simulink model "HvacMdlAirTDistbnStatFildFrnt"  (R2022b)
settings: Solver=FixedStepDiscrete  StartTime=0.0  StopTime=10.0  FixedStep=0.2  RelTol=1e-3
settings Model: Creator=blue.ge  LastModifiedBy=blue.ge
settings DataIOCC: SaveFormat=StructureWithTime
settings OptimizationCC: DefaultParameterBehavior=Inlined  EnhancedBackFolding=on  PassReuseOutputArgsAs=Individual arguments  ZeroExternalMemoryAtStartup=off  ZeroInternalMemoryAtStartup=off  OptimizeBlockOrder=speed  DifferentSizesBufferReuse=on  OptimizationCustomize=off  EfficientTunableParamExpr=on
settings DebuggingCC: ParameterTunabilityLossMsg=error
settings ModelReferenceCC: ModelReferenceNumInstancesAllowed=Single
settings SFSimCC: MATLABDynamicMemAlloc=off
settings RTWCC: Description=AUTOSAR  SystemTargetFile=autosar.tlc  GenCodeOnly=on  TemplateMakefile=ert_default_tmf
settings CodeAppCC: ForceParamTrailComments=on  IgnoreCustomStorageClasses=off  OperatorAnnotations=on  SimulinkDataObjDesc=on  SFDataObjDesc=on  InsertBlockDesc=on
settings STFCustomTargetCC: TargetLangStandard=C99 (ISO)  MultiwordLength=256  ParMdlRefBuildCompliant=on  CompOptLevelCompliant=on  IncludeMdlTerminateFcn=off  CombineOutputUpdateFcns=on  SuppressErrorStatus=on  ERTCustomFileBanners=on  SupportNonFinite=off  SupportComplex=off  SupportContinuousTime=off  SupportNonInlinedSFcns=off  RemoveResetFunc=on  MATLABClassNameForMDSCustomization=Simulink.SoftwareTarget.ERTCustomization  AutosarCompliant=on  UseToolchainInfoCompliant=on  LookupTableObjectStructAxisOrder=2,1,3,4,...  LUTObjectStructOrderEvenSpacing=Size,Table,Breakpoints  SystemTargetFile=autosar.tlc
settings ERTTargetCC: TargetLangStandard=C99 (ISO)  ConcurrentExecutionCompliant=off  IncludeMdlTerminateFcn=off  SuppressErrorStatus=on  SupportNonFinite=off  SupportComplex=off  ModelStepFunctionPrototypeControlCompliant=off  CPPClassGenCompliant=off  AutosarCompliant=on  LookupTableObjectStructAxisOrder=2,1,3,4,...  LUTObjectStructOrderEvenSpacing=Size,Table,Breakpoints  InlinedParameterPlacement=NonHierarchical  ERTCustomFileTemplate=example_file_process.tlc

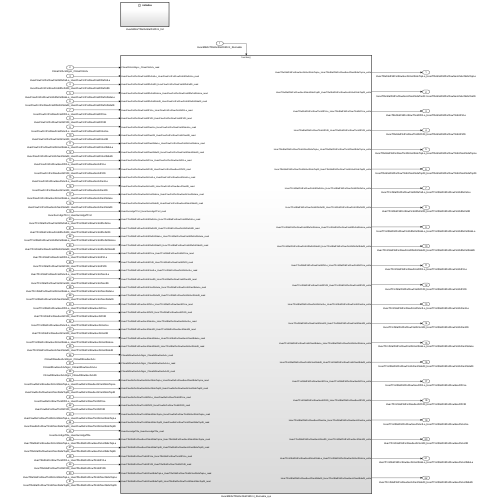
[thumbnail: Simulink's stored preview of the top-level diagram]
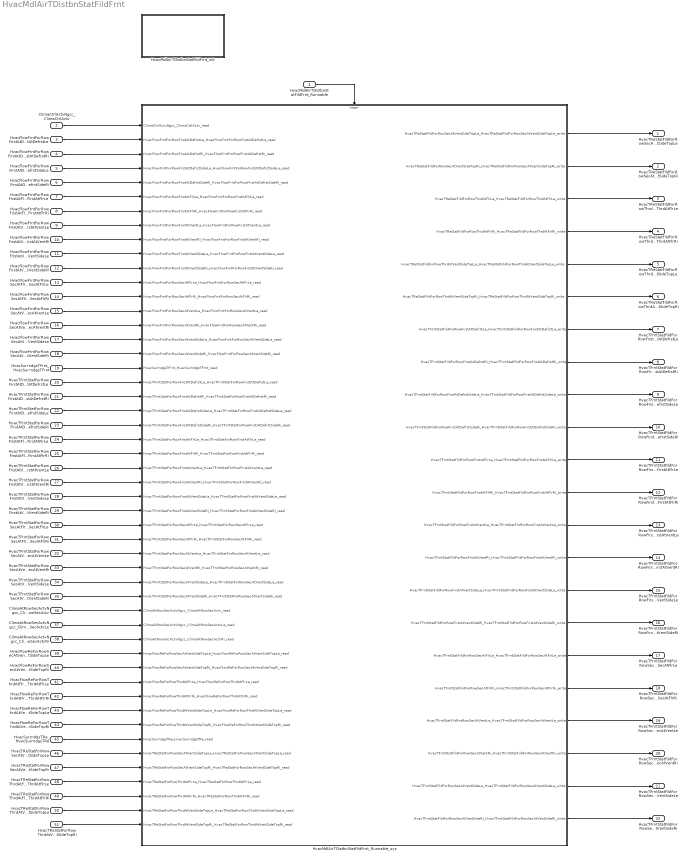
== system HvacMdlAirTDistbnStatFildFrnt ==
  HvacMdlAirTDistbnStatFildFrnt_Runnable : Inport  SampleTime=0.2  OutputFunctionCall=on
  ClimaCtrlActvNgcc_ClimaCtrlActv : Inport  Port=2  OutDataTypeStr=Boolean  PortDimensions=1
  HvacFlowFrntForRowFirstAtDefrstLe_HvacFlowFrntForRowFirstAtDefrstLe : Inport  Port=3  OutDataTypeStr=AirFlow30  PortDimensions=1
  HvacFlowFrntForRowFirstAtDefrstRi_HvacFlowFrntForRowFirstAtDefrstRi : Inport  Port=4  OutDataTypeStr=AirFlow30  PortDimensions=1
  HvacFlowFrntForRowFirstAtDefrstSideLe_HvacFlowFrntForRowFirstAtDefrstSideLe : Inport  Port=5  OutDataTypeStr=AirFlow30  PortDimensions=1
  HvacFlowFrntForRowFirstAtDefrstSideRi_HvacFlowFrntForRowFirstAtDefrstSideRi : Inport  Port=6  OutDataTypeStr=AirFlow30  PortDimensions=1
  HvacFlowFrntForRowFirstAtFlrLe_HvacFlowFrntForRowFirstAtFlrLe : Inport  Port=7  OutDataTypeStr=AirFlow30  PortDimensions=1
  HvacFlowFrntForRowFirstAtFlrRi_HvacFlowFrntForRowFirstAtFlrRi : Inport  Port=8  OutDataTypeStr=AirFlow30  PortDimensions=1
  HvacFlowFrntForRowFirstAtVentLe_HvacFlowFrntForRowFirstAtVentLe : Inport  Port=9  OutDataTypeStr=AirFlow30  PortDimensions=1
  HvacFlowFrntForRowFirstAtVentRi_HvacFlowFrntForRowFirstAtVentRi : Inport  Port=10  OutDataTypeStr=AirFlow30  PortDimensions=1
  HvacFlowFrntForRowFirstAtVentSideLe_HvacFlowFrntForRowFirstAtVentSideLe : Inport  Port=11  OutDataTypeStr=AirFlow30  PortDimensions=1
  HvacFlowFrntForRowFirstAtVentSideRi_HvacFlowFrntForRowFirstAtVentSideRi : Inport  Port=12  OutDataTypeStr=AirFlow30  PortDimensions=1
  HvacFlowFrntForRowSecAtFlrLe_HvacFlowFrntForRowSecAtFlrLe : Inport  Port=13  OutDataTypeStr=AirFlow30  PortDimensions=1
  HvacFlowFrntForRowSecAtFlrRi_HvacFlowFrntForRowSecAtFlrRi : Inport  Port=14  OutDataTypeStr=AirFlow30  PortDimensions=1
  HvacFlowFrntForRowSecAtVentLe_HvacFlowFrntForRowSecAtVentLe : Inport  Port=15  OutDataTypeStr=AirFlow30  PortDimensions=1
  HvacFlowFrntForRowSecAtVentRi_HvacFlowFrntForRowSecAtVentRi : Inport  Port=16  OutDataTypeStr=AirFlow30  PortDimensions=1
  HvacFlowFrntForRowSecAtVentSideLe_HvacFlowFrntForRowSecAtVentSideLe : Inport  Port=17  OutDataTypeStr=AirFlow30  PortDimensions=1
  HvacFlowFrntForRowSecAtVentSideRi_HvacFlowFrntForRowSecAtVentSideRi : Inport  Port=18  OutDataTypeStr=AirFlow30  PortDimensions=1
  HvacSurrndgsTFrnt_HvacSurrndgsTFrnt : Inport  Port=19  OutDataTypeStr=T30  PortDimensions=1
  HvacTFrntStatForRowFirstAtDefrstLe_HvacTFrntStatForRowFirstAtDefrstLe : Inport  Port=20  OutDataTypeStr=T30  PortDimensions=1
  HvacTFrntStatForRowFirstAtDefrstRi_HvacTFrntStatForRowFirstAtDefrstRi : Inport  Port=21  OutDataTypeStr=T30  PortDimensions=1
  HvacTFrntStatForRowFirstAtDefrstSideLe_HvacTFrntStatForRowFirstAtDefrstSideLe : Inport  Port=22  OutDataTypeStr=T30  PortDimensions=1
  HvacTFrntStatForRowFirstAtDefrstSideRi_HvacTFrntStatForRowFirstAtDefrstSideRi : Inport  Port=23  OutDataTypeStr=T30  PortDimensions=1
  HvacTFrntStatForRowFirstAtFlrLe_HvacTFrntStatForRowFirstAtFlrLe : Inport  Port=24  OutDataTypeStr=T30  PortDimensions=1
  HvacTFrntStatForRowFirstAtFlrRi_HvacTFrntStatForRowFirstAtFlrRi : Inport  Port=25  OutDataTypeStr=T30  PortDimensions=1
  HvacTFrntStatForRowFirstAtVentLe_HvacTFrntStatForRowFirstAtVentLe : Inport  Port=26  OutDataTypeStr=T30  PortDimensions=1
  HvacTFrntStatForRowFirstAtVentRi_HvacTFrntStatForRowFirstAtVentRi : Inport  Port=27  OutDataTypeStr=T30  PortDimensions=1
  HvacTFrntStatForRowFirstAtVentSideLe_HvacTFrntStatForRowFirstAtVentSideLe : Inport  Port=28  OutDataTypeStr=T30  PortDimensions=1
  HvacTFrntStatForRowFirstAtVentSideRi_HvacTFrntStatForRowFirstAtVentSideRi : Inport  Port=29  OutDataTypeStr=T30  PortDimensions=1
  HvacTFrntStatForRowSecAtFlrLe_HvacTFrntStatForRowSecAtFlrLe : Inport  Port=30  OutDataTypeStr=T30  PortDimensions=1
  HvacTFrntStatForRowSecAtFlrRi_HvacTFrntStatForRowSecAtFlrRi : Inport  Port=31  OutDataTypeStr=T30  PortDimensions=1
  HvacTFrntStatForRowSecAtVentLe_HvacTFrntStatForRowSecAtVentLe : Inport  Port=32  OutDataTypeStr=T30  PortDimensions=1
  HvacTFrntStatForRowSecAtVentRi_HvacTFrntStatForRowSecAtVentRi : Inport  Port=33  OutDataTypeStr=T30  PortDimensions=1
  HvacTFrntStatForRowSecAtVentSideLe_HvacTFrntStatForRowSecAtVentSideLe : Inport  Port=34  OutDataTypeStr=T30  PortDimensions=1
  HvacTFrntStatForRowSecAtVentSideRi_HvacTFrntStatForRowSecAtVentSideRi : Inport  Port=35  OutDataTypeStr=T30  PortDimensions=1
  ClimaAtRowSecActvNgcc_ClimaAtRowSecActv : Inport  Port=36  OutDataTypeStr=Boolean  PortDimensions=1
  ClimaAtRowSecActvNgcc_ClimaAtRowSecActvLe : Inport  Port=37  OutDataTypeStr=Boolean  PortDimensions=1
  ClimaAtRowSecActvNgcc_ClimaAtRowSecActvRi : Inport  Port=38  OutDataTypeStr=Boolean  PortDimensions=1
  HvacFlowReForRowSecAtVentSideTopLe_HvacFlowReForRowSecAtVentSideTopLe : Inport  Port=39  OutDataTypeStr=AirFlow30  PortDimensions=1
  HvacFlowReForRowSecAtVentSideTopRi_HvacFlowReForRowSecAtVentSideTopRi : Inport  Port=40  OutDataTypeStr=AirFlow30  PortDimensions=1
  HvacFlowReForRowThrdAtFlrLe_HvacFlowReForRowThrdAtFlrLe : Inport  Port=41  OutDataTypeStr=AirFlow30  PortDimensions=1
  HvacFlowReForRowThrdAtFlrRi_HvacFlowReForRowThrdAtFlrRi : Inport  Port=42  OutDataTypeStr=AirFlow30  PortDimensions=1
  HvacFlowReForRowThrdAtVentSideTopLe_HvacFlowReForRowThrdAtVentSideTopLe : Inport  Port=43  OutDataTypeStr=AirFlow30  PortDimensions=1
  HvacFlowReForRowThrdAtVentSideTopRi_HvacFlowReForRowThrdAtVentSideTopRi : Inport  Port=44  OutDataTypeStr=AirFlow30  PortDimensions=1
  HvacSurrndgsTRe_HvacSurrndgsTRe : Inport  Port=45  OutDataTypeStr=T30  PortDimensions=1
  HvacTReStatForRowSecAtVentSideTopLe_HvacTReStatForRowSecAtVentSideTopLe : Inport  Port=46  OutDataTypeStr=T30  PortDimensions=1
  HvacTReStatForRowSecAtVentSideTopRi_HvacTReStatForRowSecAtVentSideTopRi : Inport  Port=47  OutDataTypeStr=T30  PortDimensions=1
  HvacTReStatForRowThrdAtFlrLe_HvacTReStatForRowThrdAtFlrLe : Inport  Port=48  OutDataTypeStr=T30  PortDimensions=1
  HvacTReStatForRowThrdAtFlrRi_HvacTReStatForRowThrdAtFlrRi : Inport  Port=49  OutDataTypeStr=T30  PortDimensions=1
  HvacTReStatForRowThrdAtVentSideTopLe_HvacTReStatForRowThrdAtVentSideTopLe : Inport  Port=50  OutDataTypeStr=T30  PortDimensions=1
  HvacTReStatForRowThrdAtVentSideTopRi_HvacTReStatForRowThrdAtVentSideTopRi : Inport  Port=51  OutDataTypeStr=T30  PortDimensions=1
  HvacMdlAirTDistbnStatFildFrnt_Init : Subsystem
  HvacMdlAirTDistbnStatFildFrnt_Runnable_sys : Subsystem
  HvacTReStatFildForRowSecAtVentSideTopLe_HvacTReStatFildForRowSecAtVentSideTopLe : Outport  OutDataTypeStr=T30  PortDimensions=1
  HvacTReStatFildForRowSecAtVentSideTopRi_HvacTReStatFildForRowSecAtVentSideTopRi : Outport  Port=2  OutDataTypeStr=T30  PortDimensions=1
  HvacTReStatFildForRowThrdAtFlrLe_HvacTReStatFildForRowThrdAtFlrLe : Outport  Port=3  OutDataTypeStr=T30  PortDimensions=1
  HvacTReStatFildForRowThrdAtFlrRi_HvacTReStatFildForRowThrdAtFlrRi : Outport  Port=4  OutDataTypeStr=T30  PortDimensions=1
  HvacTReStatFildForRowThrdAtVentSideTopLe_HvacTReStatFildForRowThrdAtVentSideTopLe : Outport  Port=5  OutDataTypeStr=T30  PortDimensions=1
  HvacTReStatFildForRowThrdAtVentSideTopRi_HvacTReStatFildForRowThrdAtVentSideTopRi : Outport  Port=6  OutDataTypeStr=T30  PortDimensions=1
  HvacTFrntStatFildForRowFirstAtDefrstLe_HvacTFrntStatFildForRowFirstAtDefrstLe : Outport  Port=7  OutDataTypeStr=T30  PortDimensions=1
  HvacTFrntStatFildForRowFirstAtDefrstRi_HvacTFrntStatFildForRowFirstAtDefrstRi : Outport  Port=8  OutDataTypeStr=T30  PortDimensions=1
  HvacTFrntStatFildForRowFirstAtDefrstSideLe_HvacTFrntStatFildForRowFirstAtDefrstSideLe : Outport  Port=9  OutDataTypeStr=T30  PortDimensions=1
  HvacTFrntStatFildForRowFirstAtDefrstSideRi_HvacTFrntStatFildForRowFirstAtDefrstSideRi : Outport  Port=10  OutDataTypeStr=T30  PortDimensions=1
  HvacTFrntStatFildForRowFirstAtFlrLe_HvacTFrntStatFildForRowFirstAtFlrLe : Outport  Port=11  OutDataTypeStr=T30  PortDimensions=1
  HvacTFrntStatFildForRowFirstAtFlrRi_HvacTFrntStatFildForRowFirstAtFlrRi : Outport  Port=12  OutDataTypeStr=T30  PortDimensions=1
  HvacTFrntStatFildForRowFirstAtVentLe_HvacTFrntStatFildForRowFirstAtVentLe : Outport  Port=13  OutDataTypeStr=T30  PortDimensions=1
  HvacTFrntStatFildForRowFirstAtVentRi_HvacTFrntStatFildForRowFirstAtVentRi : Outport  Port=14  OutDataTypeStr=T30  PortDimensions=1
  HvacTFrntStatFildForRowFirstAtVentSideLe_HvacTFrntStatFildForRowFirstAtVentSideLe : Outport  Port=15  OutDataTypeStr=T30  PortDimensions=1
  HvacTFrntStatFildForRowFirstAtVentSideRi_HvacTFrntStatFildForRowFirstAtVentSideRi : Outport  Port=16  OutDataTypeStr=T30  PortDimensions=1
  HvacTFrntStatFildForRowSecAtFlrLe_HvacTFrntStatFildForRowSecAtFlrLe : Outport  Port=17  OutDataTypeStr=T30  PortDimensions=1
  HvacTFrntStatFildForRowSecAtFlrRi_HvacTFrntStatFildForRowSecAtFlrRi : Outport  Port=18  OutDataTypeStr=T30  PortDimensions=1
  HvacTFrntStatFildForRowSecAtVentLe_HvacTFrntStatFildForRowSecAtVentLe : Outport  Port=19  OutDataTypeStr=T30  PortDimensions=1
  HvacTFrntStatFildForRowSecAtVentRi_HvacTFrntStatFildForRowSecAtVentRi : Outport  Port=20  OutDataTypeStr=T30  PortDimensions=1
  HvacTFrntStatFildForRowSecAtVentSideLe_HvacTFrntStatFildForRowSecAtVentSideLe : Outport  Port=21  OutDataTypeStr=T30  PortDimensions=1
  HvacTFrntStatFildForRowSecAtVentSideRi_HvacTFrntStatFildForRowSecAtVentSideRi : Outport  Port=22  OutDataTypeStr=T30  PortDimensions=1
  wires:
  ClimaCtrlActvNgcc_ClimaCtrlActv/1 -> HvacMdlAirTDistbnStatFildFrnt_Runnable_sys/1(ClimaCtrlActvNgcc_ClimaCtrlActv_read)
  HvacFlowFrntForRowFirstAtDefrstLe_HvacFlowFrntForRowFirstAtDefrstLe/1 -> HvacMdlAirTDistbnStatFildFrnt_Runnable_sys/2(HvacFlowFrntForRowFirstAtDefrstLe_HvacFlowFrntForRowFirstAtDefrstLe_read)
  HvacFlowFrntForRowFirstAtDefrstRi_HvacFlowFrntForRowFirstAtDefrstRi/1 -> HvacMdlAirTDistbnStatFildFrnt_Runnable_sys/3(HvacFlowFrntForRowFirstAtDefrstRi_HvacFlowFrntForRowFirstAtDefrstRi_read)
  HvacFlowFrntForRowFirstAtDefrstSideLe_HvacFlowFrntForRowFirstAtDefrstSideLe/1 -> HvacMdlAirTDistbnStatFildFrnt_Runnable_sys/4(HvacFlowFrntForRowFirstAtDefrstSideLe_HvacFlowFrntForRowFirstAtDefrstSideLe_read)
  HvacFlowFrntForRowFirstAtDefrstSideRi_HvacFlowFrntForRowFirstAtDefrstSideRi/1 -> HvacMdlAirTDistbnStatFildFrnt_Runnable_sys/5(HvacFlowFrntForRowFirstAtDefrstSideRi_HvacFlowFrntForRowFirstAtDefrstSideRi_read)
  HvacFlowFrntForRowFirstAtFlrLe_HvacFlowFrntForRowFirstAtFlrLe/1 -> HvacMdlAirTDistbnStatFildFrnt_Runnable_sys/6(HvacFlowFrntForRowFirstAtFlrLe_HvacFlowFrntForRowFirstAtFlrLe_read)
  HvacFlowFrntForRowFirstAtFlrRi_HvacFlowFrntForRowFirstAtFlrRi/1 -> HvacMdlAirTDistbnStatFildFrnt_Runnable_sys/7(HvacFlowFrntForRowFirstAtFlrRi_HvacFlowFrntForRowFirstAtFlrRi_read)
  HvacFlowFrntForRowFirstAtVentLe_HvacFlowFrntForRowFirstAtVentLe/1 -> HvacMdlAirTDistbnStatFildFrnt_Runnable_sys/8(HvacFlowFrntForRowFirstAtVentLe_HvacFlowFrntForRowFirstAtVentLe_read)
  HvacFlowFrntForRowFirstAtVentRi_HvacFlowFrntForRowFirstAtVentRi/1 -> HvacMdlAirTDistbnStatFildFrnt_Runnable_sys/9(HvacFlowFrntForRowFirstAtVentRi_HvacFlowFrntForRowFirstAtVentRi_read)
  HvacFlowFrntForRowFirstAtVentSideLe_HvacFlowFrntForRowFirstAtVentSideLe/1 -> HvacMdlAirTDistbnStatFildFrnt_Runnable_sys/10(HvacFlowFrntForRowFirstAtVentSideLe_HvacFlowFrntForRowFirstAtVentSideLe_read)
  HvacFlowFrntForRowFirstAtVentSideRi_HvacFlowFrntForRowFirstAtVentSideRi/1 -> HvacMdlAirTDistbnStatFildFrnt_Runnable_sys/11(HvacFlowFrntForRowFirstAtVentSideRi_HvacFlowFrntForRowFirstAtVentSideRi_read)
  HvacFlowFrntForRowSecAtFlrLe_HvacFlowFrntForRowSecAtFlrLe/1 -> HvacMdlAirTDistbnStatFildFrnt_Runnable_sys/12(HvacFlowFrntForRowSecAtFlrLe_HvacFlowFrntForRowSecAtFlrLe_read)
  HvacFlowFrntForRowSecAtFlrRi_HvacFlowFrntForRowSecAtFlrRi/1 -> HvacMdlAirTDistbnStatFildFrnt_Runnable_sys/13(HvacFlowFrntForRowSecAtFlrRi_HvacFlowFrntForRowSecAtFlrRi_read)
  HvacFlowFrntForRowSecAtVentLe_HvacFlowFrntForRowSecAtVentLe/1 -> HvacMdlAirTDistbnStatFildFrnt_Runnable_sys/14(HvacFlowFrntForRowSecAtVentLe_HvacFlowFrntForRowSecAtVentLe_read)
  HvacFlowFrntForRowSecAtVentRi_HvacFlowFrntForRowSecAtVentRi/1 -> HvacMdlAirTDistbnStatFildFrnt_Runnable_sys/15(HvacFlowFrntForRowSecAtVentRi_HvacFlowFrntForRowSecAtVentRi_read)
  HvacFlowFrntForRowSecAtVentSideLe_HvacFlowFrntForRowSecAtVentSideLe/1 -> HvacMdlAirTDistbnStatFildFrnt_Runnable_sys/16(HvacFlowFrntForRowSecAtVentSideLe_HvacFlowFrntForRowSecAtVentSideLe_read)
  HvacFlowFrntForRowSecAtVentSideRi_HvacFlowFrntForRowSecAtVentSideRi/1 -> HvacMdlAirTDistbnStatFildFrnt_Runnable_sys/17(HvacFlowFrntForRowSecAtVentSideRi_HvacFlowFrntForRowSecAtVentSideRi_read)
  HvacSurrndgsTFrnt_HvacSurrndgsTFrnt/1 -> HvacMdlAirTDistbnStatFildFrnt_Runnable_sys/18(HvacSurrndgsTFrnt_HvacSurrndgsTFrnt_read)
  HvacTFrntStatForRowFirstAtDefrstLe_HvacTFrntStatForRowFirstAtDefrstLe/1 -> HvacMdlAirTDistbnStatFildFrnt_Runnable_sys/19(HvacTFrntStatForRowFirstAtDefrstLe_HvacTFrntStatForRowFirstAtDefrstLe_read)
  HvacTFrntStatForRowFirstAtDefrstRi_HvacTFrntStatForRowFirstAtDefrstRi/1 -> HvacMdlAirTDistbnStatFildFrnt_Runnable_sys/20(HvacTFrntStatForRowFirstAtDefrstRi_HvacTFrntStatForRowFirstAtDefrstRi_read)
  HvacTFrntStatForRowFirstAtDefrstSideLe_HvacTFrntStatForRowFirstAtDefrstSideLe/1 -> HvacMdlAirTDistbnStatFildFrnt_Runnable_sys/21(HvacTFrntStatForRowFirstAtDefrstSideLe_HvacTFrntStatForRowFirstAtDefrstSideLe_read)
  HvacTFrntStatForRowFirstAtDefrstSideRi_HvacTFrntStatForRowFirstAtDefrstSideRi/1 -> HvacMdlAirTDistbnStatFildFrnt_Runnable_sys/22(HvacTFrntStatForRowFirstAtDefrstSideRi_HvacTFrntStatForRowFirstAtDefrstSideRi_read)
  HvacTFrntStatForRowFirstAtFlrLe_HvacTFrntStatForRowFirstAtFlrLe/1 -> HvacMdlAirTDistbnStatFildFrnt_Runnable_sys/23(HvacTFrntStatForRowFirstAtFlrLe_HvacTFrntStatForRowFirstAtFlrLe_read)
  HvacTFrntStatForRowFirstAtFlrRi_HvacTFrntStatForRowFirstAtFlrRi/1 -> HvacMdlAirTDistbnStatFildFrnt_Runnable_sys/24(HvacTFrntStatForRowFirstAtFlrRi_HvacTFrntStatForRowFirstAtFlrRi_read)
  HvacTFrntStatForRowFirstAtVentLe_HvacTFrntStatForRowFirstAtVentLe/1 -> HvacMdlAirTDistbnStatFildFrnt_Runnable_sys/25(HvacTFrntStatForRowFirstAtVentLe_HvacTFrntStatForRowFirstAtVentLe_read)
  HvacTFrntStatForRowFirstAtVentRi_HvacTFrntStatForRowFirstAtVentRi/1 -> HvacMdlAirTDistbnStatFildFrnt_Runnable_sys/26(HvacTFrntStatForRowFirstAtVentRi_HvacTFrntStatForRowFirstAtVentRi_read)
  HvacTFrntStatForRowFirstAtVentSideLe_HvacTFrntStatForRowFirstAtVentSideLe/1 -> HvacMdlAirTDistbnStatFildFrnt_Runnable_sys/27(HvacTFrntStatForRowFirstAtVentSideLe_HvacTFrntStatForRowFirstAtVentSideLe_read)
  HvacTFrntStatForRowFirstAtVentSideRi_HvacTFrntStatForRowFirstAtVentSideRi/1 -> HvacMdlAirTDistbnStatFildFrnt_Runnable_sys/28(HvacTFrntStatForRowFirstAtVentSideRi_HvacTFrntStatForRowFirstAtVentSideRi_read)
  HvacTFrntStatForRowSecAtFlrLe_HvacTFrntStatForRowSecAtFlrLe/1 -> HvacMdlAirTDistbnStatFildFrnt_Runnable_sys/29(HvacTFrntStatForRowSecAtFlrLe_HvacTFrntStatForRowSecAtFlrLe_read)
  HvacTFrntStatForRowSecAtFlrRi_HvacTFrntStatForRowSecAtFlrRi/1 -> HvacMdlAirTDistbnStatFildFrnt_Runnable_sys/30(HvacTFrntStatForRowSecAtFlrRi_HvacTFrntStatForRowSecAtFlrRi_read)
  HvacTFrntStatForRowSecAtVentLe_HvacTFrntStatForRowSecAtVentLe/1 -> HvacMdlAirTDistbnStatFildFrnt_Runnable_sys/31(HvacTFrntStatForRowSecAtVentLe_HvacTFrntStatForRowSecAtVentLe_read)
  HvacTFrntStatForRowSecAtVentRi_HvacTFrntStatForRowSecAtVentRi/1 -> HvacMdlAirTDistbnStatFildFrnt_Runnable_sys/32(HvacTFrntStatForRowSecAtVentRi_HvacTFrntStatForRowSecAtVentRi_read)
  HvacTFrntStatForRowSecAtVentSideLe_HvacTFrntStatForRowSecAtVentSideLe/1 -> HvacMdlAirTDistbnStatFildFrnt_Runnable_sys/33(HvacTFrntStatForRowSecAtVentSideLe_HvacTFrntStatForRowSecAtVentSideLe_read)
  HvacTFrntStatForRowSecAtVentSideRi_HvacTFrntStatForRowSecAtVentSideRi/1 -> HvacMdlAirTDistbnStatFildFrnt_Runnable_sys/34(HvacTFrntStatForRowSecAtVentSideRi_HvacTFrntStatForRowSecAtVentSideRi_read)
  ClimaAtRowSecActvNgcc_ClimaAtRowSecActv/1 -> HvacMdlAirTDistbnStatFildFrnt_Runnable_sys/35(ClimaAtRowSecActvNgcc_ClimaAtRowSecActv_read)
  ClimaAtRowSecActvNgcc_ClimaAtRowSecActvLe/1 -> HvacMdlAirTDistbnStatFildFrnt_Runnable_sys/36(ClimaAtRowSecActvNgcc_ClimaAtRowSecActvLe_read)
  ClimaAtRowSecActvNgcc_ClimaAtRowSecActvRi/1 -> HvacMdlAirTDistbnStatFildFrnt_Runnable_sys/37(ClimaAtRowSecActvNgcc_ClimaAtRowSecActvRi_read)
  HvacFlowReForRowSecAtVentSideTopLe_HvacFlowReForRowSecAtVentSideTopLe/1 -> HvacMdlAirTDistbnStatFildFrnt_Runnable_sys/38(HvacFlowReForRowSecAtVentSideTopLe_HvacFlowReForRowSecAtVentSideTopLe_read)
  HvacFlowReForRowSecAtVentSideTopRi_HvacFlowReForRowSecAtVentSideTopRi/1 -> HvacMdlAirTDistbnStatFildFrnt_Runnable_sys/39(HvacFlowReForRowSecAtVentSideTopRi_HvacFlowReForRowSecAtVentSideTopRi_read)
  HvacFlowReForRowThrdAtFlrLe_HvacFlowReForRowThrdAtFlrLe/1 -> HvacMdlAirTDistbnStatFildFrnt_Runnable_sys/40(HvacFlowReForRowThrdAtFlrLe_HvacFlowReForRowThrdAtFlrLe_read)
  HvacFlowReForRowThrdAtFlrRi_HvacFlowReForRowThrdAtFlrRi/1 -> HvacMdlAirTDistbnStatFildFrnt_Runnable_sys/41(HvacFlowReForRowThrdAtFlrRi_HvacFlowReForRowThrdAtFlrRi_read)
  HvacFlowReForRowThrdAtVentSideTopLe_HvacFlowReForRowThrdAtVentSideTopLe/1 -> HvacMdlAirTDistbnStatFildFrnt_Runnable_sys/42(HvacFlowReForRowThrdAtVentSideTopLe_HvacFlowReForRowThrdAtVentSideTopLe_read)
  HvacFlowReForRowThrdAtVentSideTopRi_HvacFlowReForRowThrdAtVentSideTopRi/1 -> HvacMdlAirTDistbnStatFildFrnt_Runnable_sys/43(HvacFlowReForRowThrdAtVentSideTopRi_HvacFlowReForRowThrdAtVentSideTopRi_read)
  HvacSurrndgsTRe_HvacSurrndgsTRe/1 -> HvacMdlAirTDistbnStatFildFrnt_Runnable_sys/44(HvacSurrndgsTRe_HvacSurrndgsTRe_read)
  HvacTReStatForRowSecAtVentSideTopLe_HvacTReStatForRowSecAtVentSideTopLe/1 -> HvacMdlAirTDistbnStatFildFrnt_Runnable_sys/45(HvacTReStatForRowSecAtVentSideTopLe_HvacTReStatForRowSecAtVentSideTopLe_read)
  HvacTReStatForRowSecAtVentSideTopRi_HvacTReStatForRowSecAtVentSideTopRi/1 -> HvacMdlAirTDistbnStatFildFrnt_Runnable_sys/46(HvacTReStatForRowSecAtVentSideTopRi_HvacTReStatForRowSecAtVentSideTopRi_read)
  HvacTReStatForRowThrdAtFlrLe_HvacTReStatForRowThrdAtFlrLe/1 -> HvacMdlAirTDistbnStatFildFrnt_Runnable_sys/47(HvacTReStatForRowThrdAtFlrLe_HvacTReStatForRowThrdAtFlrLe_read)
  HvacTReStatForRowThrdAtFlrRi_HvacTReStatForRowThrdAtFlrRi/1 -> HvacMdlAirTDistbnStatFildFrnt_Runnable_sys/48(HvacTReStatForRowThrdAtFlrRi_HvacTReStatForRowThrdAtFlrRi_read)
  HvacTReStatForRowThrdAtVentSideTopLe_HvacTReStatForRowThrdAtVentSideTopLe/1 -> HvacMdlAirTDistbnStatFildFrnt_Runnable_sys/49(HvacTReStatForRowThrdAtVentSideTopLe_HvacTReStatForRowThrdAtVentSideTopLe_read)
  HvacTReStatForRowThrdAtVentSideTopRi_HvacTReStatForRowThrdAtVentSideTopRi/1 -> HvacMdlAirTDistbnStatFildFrnt_Runnable_sys/50(HvacTReStatForRowThrdAtVentSideTopRi_HvacTReStatForRowThrdAtVentSideTopRi_read)
  HvacMdlAirTDistbnStatFildFrnt_Runnable_sys/1(HvacTReStatFildForRowSecAtVentSideTopLe_HvacTReStatFildForRowSecAtVentSideTopLe_write) -> HvacTReStatFildForRowSecAtVentSideTopLe_HvacTReStatFildForRowSecAtVentSideTopLe/1
  HvacMdlAirTDistbnStatFildFrnt_Runnable_sys/2(HvacTReStatFildForRowSecAtVentSideTopRi_HvacTReStatFildForRowSecAtVentSideTopRi_write) -> HvacTReStatFildForRowSecAtVentSideTopRi_HvacTReStatFildForRowSecAtVentSideTopRi/1
  HvacMdlAirTDistbnStatFildFrnt_Runnable_sys/3(HvacTReStatFildForRowThrdAtFlrLe_HvacTReStatFildForRowThrdAtFlrLe_write) -> HvacTReStatFildForRowThrdAtFlrLe_HvacTReStatFildForRowThrdAtFlrLe/1
  HvacMdlAirTDistbnStatFildFrnt_Runnable_sys/4(HvacTReStatFildForRowThrdAtFlrRi_HvacTReStatFildForRowThrdAtFlrRi_write) -> HvacTReStatFildForRowThrdAtFlrRi_HvacTReStatFildForRowThrdAtFlrRi/1
  HvacMdlAirTDistbnStatFildFrnt_Runnable_sys/5(HvacTReStatFildForRowThrdAtVentSideTopLe_HvacTReStatFildForRowThrdAtVentSideTopLe_write) -> HvacTReStatFildForRowThrdAtVentSideTopLe_HvacTReStatFildForRowThrdAtVentSideTopLe/1
  HvacMdlAirTDistbnStatFildFrnt_Runnable_sys/6(HvacTReStatFildForRowThrdAtVentSideTopRi_HvacTReStatFildForRowThrdAtVentSideTopRi_write) -> HvacTReStatFildForRowThrdAtVentSideTopRi_HvacTReStatFildForRowThrdAtVentSideTopRi/1
  HvacMdlAirTDistbnStatFildFrnt_Runnable_sys/7(HvacTFrntStatFildForRowFirstAtDefrstLe_HvacTFrntStatFildForRowFirstAtDefrstLe_write) -> HvacTFrntStatFildForRowFirstAtDefrstLe_HvacTFrntStatFildForRowFirstAtDefrstLe/1
  HvacMdlAirTDistbnStatFildFrnt_Runnable_sys/8(HvacTFrntStatFildForRowFirstAtDefrstRi_HvacTFrntStatFildForRowFirstAtDefrstRi_write) -> HvacTFrntStatFildForRowFirstAtDefrstRi_HvacTFrntStatFildForRowFirstAtDefrstRi/1
  HvacMdlAirTDistbnStatFildFrnt_Runnable_sys/9(HvacTFrntStatFildForRowFirstAtDefrstSideLe_HvacTFrntStatFildForRowFirstAtDefrstSideLe_write) -> HvacTFrntStatFildForRowFirstAtDefrstSideLe_HvacTFrntStatFildForRowFirstAtDefrstSideLe/1
  HvacMdlAirTDistbnStatFildFrnt_Runnable_sys/10(HvacTFrntStatFildForRowFirstAtDefrstSideRi_HvacTFrntStatFildForRowFirstAtDefrstSideRi_write) -> HvacTFrntStatFildForRowFirstAtDefrstSideRi_HvacTFrntStatFildForRowFirstAtDefrstSideRi/1
  HvacMdlAirTDistbnStatFildFrnt_Runnable_sys/11(HvacTFrntStatFildForRowFirstAtFlrLe_HvacTFrntStatFildForRowFirstAtFlrLe_write) -> HvacTFrntStatFildForRowFirstAtFlrLe_HvacTFrntStatFildForRowFirstAtFlrLe/1
  HvacMdlAirTDistbnStatFildFrnt_Runnable_sys/12(HvacTFrntStatFildForRowFirstAtFlrRi_HvacTFrntStatFildForRowFirstAtFlrRi_write) -> HvacTFrntStatFildForRowFirstAtFlrRi_HvacTFrntStatFildForRowFirstAtFlrRi/1
  HvacMdlAirTDistbnStatFildFrnt_Runnable_sys/13(HvacTFrntStatFildForRowFirstAtVentLe_HvacTFrntStatFildForRowFirstAtVentLe_write) -> HvacTFrntStatFildForRowFirstAtVentLe_HvacTFrntStatFildForRowFirstAtVentLe/1
  HvacMdlAirTDistbnStatFildFrnt_Runnable_sys/14(HvacTFrntStatFildForRowFirstAtVentRi_HvacTFrntStatFildForRowFirstAtVentRi_write) -> HvacTFrntStatFildForRowFirstAtVentRi_HvacTFrntStatFildForRowFirstAtVentRi/1
  HvacMdlAirTDistbnStatFildFrnt_Runnable_sys/15(HvacTFrntStatFildForRowFirstAtVentSideLe_HvacTFrntStatFildForRowFirstAtVentSideLe_write) -> HvacTFrntStatFildForRowFirstAtVentSideLe_HvacTFrntStatFildForRowFirstAtVentSideLe/1
  HvacMdlAirTDistbnStatFildFrnt_Runnable_sys/16(HvacTFrntStatFildForRowFirstAtVentSideRi_HvacTFrntStatFildForRowFirstAtVentSideRi_write) -> HvacTFrntStatFildForRowFirstAtVentSideRi_HvacTFrntStatFildForRowFirstAtVentSideRi/1
  HvacMdlAirTDistbnStatFildFrnt_Runnable_sys/17(HvacTFrntStatFildForRowSecAtFlrLe_HvacTFrntStatFildForRowSecAtFlrLe_write) -> HvacTFrntStatFildForRowSecAtFlrLe_HvacTFrntStatFildForRowSecAtFlrLe/1
  HvacMdlAirTDistbnStatFildFrnt_Runnable_sys/18(HvacTFrntStatFildForRowSecAtFlrRi_HvacTFrntStatFildForRowSecAtFlrRi_write) -> HvacTFrntStatFildForRowSecAtFlrRi_HvacTFrntStatFildForRowSecAtFlrRi/1
  HvacMdlAirTDistbnStatFildFrnt_Runnable_sys/19(HvacTFrntStatFildForRowSecAtVentLe_HvacTFrntStatFildForRowSecAtVentLe_write) -> HvacTFrntStatFildForRowSecAtVentLe_HvacTFrntStatFildForRowSecAtVentLe/1
  HvacMdlAirTDistbnStatFildFrnt_Runnable_sys/20(HvacTFrntStatFildForRowSecAtVentRi_HvacTFrntStatFildForRowSecAtVentRi_write) -> HvacTFrntStatFildForRowSecAtVentRi_HvacTFrntStatFildForRowSecAtVentRi/1
  HvacMdlAirTDistbnStatFildFrnt_Runnable_sys/21(HvacTFrntStatFildForRowSecAtVentSideLe_HvacTFrntStatFildForRowSecAtVentSideLe_write) -> HvacTFrntStatFildForRowSecAtVentSideLe_HvacTFrntStatFildForRowSecAtVentSideLe/1
  HvacMdlAirTDistbnStatFildFrnt_Runnable_sys/22(HvacTFrntStatFildForRowSecAtVentSideRi_HvacTFrntStatFildForRowSecAtVentSideRi_write) -> HvacTFrntStatFildForRowSecAtVentSideRi_HvacTFrntStatFildForRowSecAtVentSideRi/1
  HvacMdlAirTDistbnStatFildFrnt_Runnable/1 -> HvacMdlAirTDistbnStatFildFrnt_Runnable_sys/Trigger

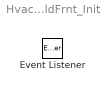
== system HvacMdlAirTDistbnStatFildFrnt/HvacMdlAirTDistbnStatFildFrnt_Init ==
  Event Listener : EventListener  EventName=reset

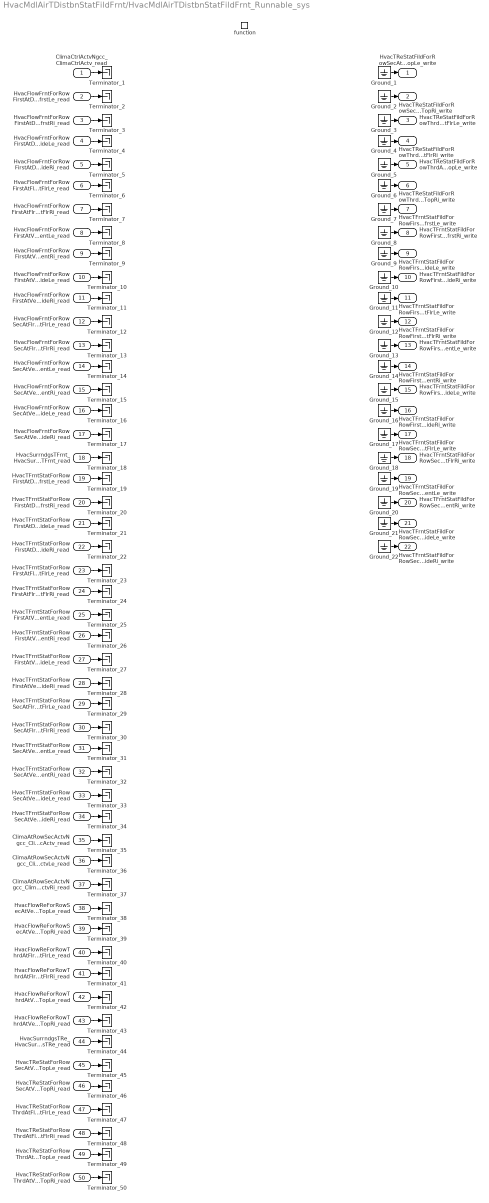
== system HvacMdlAirTDistbnStatFildFrnt/HvacMdlAirTDistbnStatFildFrnt_Runnable_sys ==
  ClimaCtrlActvNgcc_ClimaCtrlActv_read : Inport
  HvacFlowFrntForRowFirstAtDefrstLe_HvacFlowFrntForRowFirstAtDefrstLe_read : Inport  Port=2
  HvacFlowFrntForRowFirstAtDefrstRi_HvacFlowFrntForRowFirstAtDefrstRi_read : Inport  Port=3
  HvacFlowFrntForRowFirstAtDefrstSideLe_HvacFlowFrntForRowFirstAtDefrstSideLe_read : Inport  Port=4
  HvacFlowFrntForRowFirstAtDefrstSideRi_HvacFlowFrntForRowFirstAtDefrstSideRi_read : Inport  Port=5
  HvacFlowFrntForRowFirstAtFlrLe_HvacFlowFrntForRowFirstAtFlrLe_read : Inport  Port=6
  HvacFlowFrntForRowFirstAtFlrRi_HvacFlowFrntForRowFirstAtFlrRi_read : Inport  Port=7
  HvacFlowFrntForRowFirstAtVentLe_HvacFlowFrntForRowFirstAtVentLe_read : Inport  Port=8
  HvacFlowFrntForRowFirstAtVentRi_HvacFlowFrntForRowFirstAtVentRi_read : Inport  Port=9
  HvacFlowFrntForRowFirstAtVentSideLe_HvacFlowFrntForRowFirstAtVentSideLe_read : Inport  Port=10
  HvacFlowFrntForRowFirstAtVentSideRi_HvacFlowFrntForRowFirstAtVentSideRi_read : Inport  Port=11
  HvacFlowFrntForRowSecAtFlrLe_HvacFlowFrntForRowSecAtFlrLe_read : Inport  Port=12
  HvacFlowFrntForRowSecAtFlrRi_HvacFlowFrntForRowSecAtFlrRi_read : Inport  Port=13
  HvacFlowFrntForRowSecAtVentLe_HvacFlowFrntForRowSecAtVentLe_read : Inport  Port=14
  HvacFlowFrntForRowSecAtVentRi_HvacFlowFrntForRowSecAtVentRi_read : Inport  Port=15
  HvacFlowFrntForRowSecAtVentSideLe_HvacFlowFrntForRowSecAtVentSideLe_read : Inport  Port=16
  HvacFlowFrntForRowSecAtVentSideRi_HvacFlowFrntForRowSecAtVentSideRi_read : Inport  Port=17
  HvacSurrndgsTFrnt_HvacSurrndgsTFrnt_read : Inport  Port=18
  HvacTFrntStatForRowFirstAtDefrstLe_HvacTFrntStatForRowFirstAtDefrstLe_read : Inport  Port=19
  HvacTFrntStatForRowFirstAtDefrstRi_HvacTFrntStatForRowFirstAtDefrstRi_read : Inport  Port=20
  HvacTFrntStatForRowFirstAtDefrstSideLe_HvacTFrntStatForRowFirstAtDefrstSideLe_read : Inport  Port=21
  HvacTFrntStatForRowFirstAtDefrstSideRi_HvacTFrntStatForRowFirstAtDefrstSideRi_read : Inport  Port=22
  HvacTFrntStatForRowFirstAtFlrLe_HvacTFrntStatForRowFirstAtFlrLe_read : Inport  Port=23
  HvacTFrntStatForRowFirstAtFlrRi_HvacTFrntStatForRowFirstAtFlrRi_read : Inport  Port=24
  HvacTFrntStatForRowFirstAtVentLe_HvacTFrntStatForRowFirstAtVentLe_read : Inport  Port=25
  HvacTFrntStatForRowFirstAtVentRi_HvacTFrntStatForRowFirstAtVentRi_read : Inport  Port=26
  HvacTFrntStatForRowFirstAtVentSideLe_HvacTFrntStatForRowFirstAtVentSideLe_read : Inport  Port=27
  HvacTFrntStatForRowFirstAtVentSideRi_HvacTFrntStatForRowFirstAtVentSideRi_read : Inport  Port=28
  HvacTFrntStatForRowSecAtFlrLe_HvacTFrntStatForRowSecAtFlrLe_read : Inport  Port=29
  HvacTFrntStatForRowSecAtFlrRi_HvacTFrntStatForRowSecAtFlrRi_read : Inport  Port=30
  HvacTFrntStatForRowSecAtVentLe_HvacTFrntStatForRowSecAtVentLe_read : Inport  Port=31
  HvacTFrntStatForRowSecAtVentRi_HvacTFrntStatForRowSecAtVentRi_read : Inport  Port=32
  HvacTFrntStatForRowSecAtVentSideLe_HvacTFrntStatForRowSecAtVentSideLe_read : Inport  Port=33
  HvacTFrntStatForRowSecAtVentSideRi_HvacTFrntStatForRowSecAtVentSideRi_read : Inport  Port=34
  ClimaAtRowSecActvNgcc_ClimaAtRowSecActv_read : Inport  Port=35
  ClimaAtRowSecActvNgcc_ClimaAtRowSecActvLe_read : Inport  Port=36
  ClimaAtRowSecActvNgcc_ClimaAtRowSecActvRi_read : Inport  Port=37
  HvacFlowReForRowSecAtVentSideTopLe_HvacFlowReForRowSecAtVentSideTopLe_read : Inport  Port=38
  HvacFlowReForRowSecAtVentSideTopRi_HvacFlowReForRowSecAtVentSideTopRi_read : Inport  Port=39
  HvacFlowReForRowThrdAtFlrLe_HvacFlowReForRowThrdAtFlrLe_read : Inport  Port=40
  HvacFlowReForRowThrdAtFlrRi_HvacFlowReForRowThrdAtFlrRi_read : Inport  Port=41
  HvacFlowReForRowThrdAtVentSideTopLe_HvacFlowReForRowThrdAtVentSideTopLe_read : Inport  Port=42
  HvacFlowReForRowThrdAtVentSideTopRi_HvacFlowReForRowThrdAtVentSideTopRi_read : Inport  Port=43
  HvacSurrndgsTRe_HvacSurrndgsTRe_read : Inport  Port=44
  HvacTReStatForRowSecAtVentSideTopLe_HvacTReStatForRowSecAtVentSideTopLe_read : Inport  Port=45
  HvacTReStatForRowSecAtVentSideTopRi_HvacTReStatForRowSecAtVentSideTopRi_read : Inport  Port=46
  HvacTReStatForRowThrdAtFlrLe_HvacTReStatForRowThrdAtFlrLe_read : Inport  Port=47
  HvacTReStatForRowThrdAtFlrRi_HvacTReStatForRowThrdAtFlrRi_read : Inport  Port=48
  HvacTReStatForRowThrdAtVentSideTopLe_HvacTReStatForRowThrdAtVentSideTopLe_read : Inport  Port=49
  HvacTReStatForRowThrdAtVentSideTopRi_HvacTReStatForRowThrdAtVentSideTopRi_read : Inport  Port=50
  function : TriggerPort  TriggerType=function-call  VariantControl=(inherit)  SampleTimeType=periodic  SampleTime=-1
  Ground_{1..22} (22 alike) : Ground
  Terminator_{1..50} (50 alike) : Terminator
  HvacTReStatFildForRowSecAtVentSideTopLe_HvacTReStatFildForRowSecAtVentSideTopLe_write : Outport
      settings: EnsureOutportIsVirtual=on
  HvacTReStatFildForRowSecAtVentSideTopRi_HvacTReStatFildForRowSecAtVentSideTopRi_write : Outport  Port=2
      settings: EnsureOutportIsVirtual=on
  HvacTReStatFildForRowThrdAtFlrLe_HvacTReStatFildForRowThrdAtFlrLe_write : Outport  Port=3
      settings: EnsureOutportIsVirtual=on
  HvacTReStatFildForRowThrdAtFlrRi_HvacTReStatFildForRowThrdAtFlrRi_write : Outport  Port=4
      settings: EnsureOutportIsVirtual=on
  HvacTReStatFildForRowThrdAtVentSideTopLe_HvacTReStatFildForRowThrdAtVentSideTopLe_write : Outport  Port=5
      settings: EnsureOutportIsVirtual=on
  HvacTReStatFildForRowThrdAtVentSideTopRi_HvacTReStatFildForRowThrdAtVentSideTopRi_write : Outport  Port=6
      settings: EnsureOutportIsVirtual=on
  HvacTFrntStatFildForRowFirstAtDefrstLe_HvacTFrntStatFildForRowFirstAtDefrstLe_write : Outport  Port=7
      settings: EnsureOutportIsVirtual=on
  HvacTFrntStatFildForRowFirstAtDefrstRi_HvacTFrntStatFildForRowFirstAtDefrstRi_write : Outport  Port=8
      settings: EnsureOutportIsVirtual=on
  HvacTFrntStatFildForRowFirstAtDefrstSideLe_HvacTFrntStatFildForRowFirstAtDefrstSideLe_write : Outport  Port=9
      settings: EnsureOutportIsVirtual=on
  HvacTFrntStatFildForRowFirstAtDefrstSideRi_HvacTFrntStatFildForRowFirstAtDefrstSideRi_write : Outport  Port=10
      settings: EnsureOutportIsVirtual=on
  HvacTFrntStatFildForRowFirstAtFlrLe_HvacTFrntStatFildForRowFirstAtFlrLe_write : Outport  Port=11
      settings: EnsureOutportIsVirtual=on
  HvacTFrntStatFildForRowFirstAtFlrRi_HvacTFrntStatFildForRowFirstAtFlrRi_write : Outport  Port=12
      settings: EnsureOutportIsVirtual=on
  HvacTFrntStatFildForRowFirstAtVentLe_HvacTFrntStatFildForRowFirstAtVentLe_write : Outport  Port=13
      settings: EnsureOutportIsVirtual=on
  HvacTFrntStatFildForRowFirstAtVentRi_HvacTFrntStatFildForRowFirstAtVentRi_write : Outport  Port=14
      settings: EnsureOutportIsVirtual=on
  HvacTFrntStatFildForRowFirstAtVentSideLe_HvacTFrntStatFildForRowFirstAtVentSideLe_write : Outport  Port=15
      settings: EnsureOutportIsVirtual=on
  HvacTFrntStatFildForRowFirstAtVentSideRi_HvacTFrntStatFildForRowFirstAtVentSideRi_write : Outport  Port=16
      settings: EnsureOutportIsVirtual=on
  HvacTFrntStatFildForRowSecAtFlrLe_HvacTFrntStatFildForRowSecAtFlrLe_write : Outport  Port=17
      settings: EnsureOutportIsVirtual=on
  HvacTFrntStatFildForRowSecAtFlrRi_HvacTFrntStatFildForRowSecAtFlrRi_write : Outport  Port=18
      settings: EnsureOutportIsVirtual=on
  HvacTFrntStatFildForRowSecAtVentLe_HvacTFrntStatFildForRowSecAtVentLe_write : Outport  Port=19
      settings: EnsureOutportIsVirtual=on
  HvacTFrntStatFildForRowSecAtVentRi_HvacTFrntStatFildForRowSecAtVentRi_write : Outport  Port=20
      settings: EnsureOutportIsVirtual=on
  HvacTFrntStatFildForRowSecAtVentSideLe_HvacTFrntStatFildForRowSecAtVentSideLe_write : Outport  Port=21
      settings: EnsureOutportIsVirtual=on
  HvacTFrntStatFildForRowSecAtVentSideRi_HvacTFrntStatFildForRowSecAtVentSideRi_write : Outport  Port=22
      settings: EnsureOutportIsVirtual=on
  wires:
  ClimaCtrlActvNgcc_ClimaCtrlActv_read/1 -> Terminator_1/1
  HvacFlowFrntForRowFirstAtDefrstLe_HvacFlowFrntForRowFirstAtDefrstLe_read/1 -> Terminator_2/1
  HvacFlowFrntForRowFirstAtDefrstRi_HvacFlowFrntForRowFirstAtDefrstRi_read/1 -> Terminator_3/1
  HvacFlowFrntForRowFirstAtDefrstSideLe_HvacFlowFrntForRowFirstAtDefrstSideLe_read/1 -> Terminator_4/1
  HvacFlowFrntForRowFirstAtDefrstSideRi_HvacFlowFrntForRowFirstAtDefrstSideRi_read/1 -> Terminator_5/1
  HvacFlowFrntForRowFirstAtFlrLe_HvacFlowFrntForRowFirstAtFlrLe_read/1 -> Terminator_6/1
  HvacFlowFrntForRowFirstAtFlrRi_HvacFlowFrntForRowFirstAtFlrRi_read/1 -> Terminator_7/1
  HvacFlowFrntForRowFirstAtVentLe_HvacFlowFrntForRowFirstAtVentLe_read/1 -> Terminator_8/1
  HvacFlowFrntForRowFirstAtVentRi_HvacFlowFrntForRowFirstAtVentRi_read/1 -> Terminator_9/1
  HvacFlowFrntForRowFirstAtVentSideLe_HvacFlowFrntForRowFirstAtVentSideLe_read/1 -> Terminator_10/1
  HvacFlowFrntForRowFirstAtVentSideRi_HvacFlowFrntForRowFirstAtVentSideRi_read/1 -> Terminator_11/1
  HvacFlowFrntForRowSecAtFlrLe_HvacFlowFrntForRowSecAtFlrLe_read/1 -> Terminator_12/1
  HvacFlowFrntForRowSecAtFlrRi_HvacFlowFrntForRowSecAtFlrRi_read/1 -> Terminator_13/1
  HvacFlowFrntForRowSecAtVentLe_HvacFlowFrntForRowSecAtVentLe_read/1 -> Terminator_14/1
  HvacFlowFrntForRowSecAtVentRi_HvacFlowFrntForRowSecAtVentRi_read/1 -> Terminator_15/1
  HvacFlowFrntForRowSecAtVentSideLe_HvacFlowFrntForRowSecAtVentSideLe_read/1 -> Terminator_16/1
  HvacFlowFrntForRowSecAtVentSideRi_HvacFlowFrntForRowSecAtVentSideRi_read/1 -> Terminator_17/1
  HvacSurrndgsTFrnt_HvacSurrndgsTFrnt_read/1 -> Terminator_18/1
  HvacTFrntStatForRowFirstAtDefrstLe_HvacTFrntStatForRowFirstAtDefrstLe_read/1 -> Terminator_19/1
  HvacTFrntStatForRowFirstAtDefrstRi_HvacTFrntStatForRowFirstAtDefrstRi_read/1 -> Terminator_20/1
  HvacTFrntStatForRowFirstAtDefrstSideLe_HvacTFrntStatForRowFirstAtDefrstSideLe_read/1 -> Terminator_21/1
  HvacTFrntStatForRowFirstAtDefrstSideRi_HvacTFrntStatForRowFirstAtDefrstSideRi_read/1 -> Terminator_22/1
  HvacTFrntStatForRowFirstAtFlrLe_HvacTFrntStatForRowFirstAtFlrLe_read/1 -> Terminator_23/1
  HvacTFrntStatForRowFirstAtFlrRi_HvacTFrntStatForRowFirstAtFlrRi_read/1 -> Terminator_24/1
  HvacTFrntStatForRowFirstAtVentLe_HvacTFrntStatForRowFirstAtVentLe_read/1 -> Terminator_25/1
  HvacTFrntStatForRowFirstAtVentRi_HvacTFrntStatForRowFirstAtVentRi_read/1 -> Terminator_26/1
  HvacTFrntStatForRowFirstAtVentSideLe_HvacTFrntStatForRowFirstAtVentSideLe_read/1 -> Terminator_27/1
  HvacTFrntStatForRowFirstAtVentSideRi_HvacTFrntStatForRowFirstAtVentSideRi_read/1 -> Terminator_28/1
  HvacTFrntStatForRowSecAtFlrLe_HvacTFrntStatForRowSecAtFlrLe_read/1 -> Terminator_29/1
  HvacTFrntStatForRowSecAtFlrRi_HvacTFrntStatForRowSecAtFlrRi_read/1 -> Terminator_30/1
  HvacTFrntStatForRowSecAtVentLe_HvacTFrntStatForRowSecAtVentLe_read/1 -> Terminator_31/1
  HvacTFrntStatForRowSecAtVentRi_HvacTFrntStatForRowSecAtVentRi_read/1 -> Terminator_32/1
  HvacTFrntStatForRowSecAtVentSideLe_HvacTFrntStatForRowSecAtVentSideLe_read/1 -> Terminator_33/1
  HvacTFrntStatForRowSecAtVentSideRi_HvacTFrntStatForRowSecAtVentSideRi_read/1 -> Terminator_34/1
  ClimaAtRowSecActvNgcc_ClimaAtRowSecActv_read/1 -> Terminator_35/1
  ClimaAtRowSecActvNgcc_ClimaAtRowSecActvLe_read/1 -> Terminator_36/1
  ClimaAtRowSecActvNgcc_ClimaAtRowSecActvRi_read/1 -> Terminator_37/1
  HvacFlowReForRowSecAtVentSideTopLe_HvacFlowReForRowSecAtVentSideTopLe_read/1 -> Terminator_38/1
  HvacFlowReForRowSecAtVentSideTopRi_HvacFlowReForRowSecAtVentSideTopRi_read/1 -> Terminator_39/1
  HvacFlowReForRowThrdAtFlrLe_HvacFlowReForRowThrdAtFlrLe_read/1 -> Terminator_40/1
  HvacFlowReForRowThrdAtFlrRi_HvacFlowReForRowThrdAtFlrRi_read/1 -> Terminator_41/1
  HvacFlowReForRowThrdAtVentSideTopLe_HvacFlowReForRowThrdAtVentSideTopLe_read/1 -> Terminator_42/1
  HvacFlowReForRowThrdAtVentSideTopRi_HvacFlowReForRowThrdAtVentSideTopRi_read/1 -> Terminator_43/1
  HvacSurrndgsTRe_HvacSurrndgsTRe_read/1 -> Terminator_44/1
  HvacTReStatForRowSecAtVentSideTopLe_HvacTReStatForRowSecAtVentSideTopLe_read/1 -> Terminator_45/1
  HvacTReStatForRowSecAtVentSideTopRi_HvacTReStatForRowSecAtVentSideTopRi_read/1 -> Terminator_46/1
  HvacTReStatForRowThrdAtFlrLe_HvacTReStatForRowThrdAtFlrLe_read/1 -> Terminator_47/1
  HvacTReStatForRowThrdAtFlrRi_HvacTReStatForRowThrdAtFlrRi_read/1 -> Terminator_48/1
  HvacTReStatForRowThrdAtVentSideTopLe_HvacTReStatForRowThrdAtVentSideTopLe_read/1 -> Terminator_49/1
  HvacTReStatForRowThrdAtVentSideTopRi_HvacTReStatForRowThrdAtVentSideTopRi_read/1 -> Terminator_50/1
  Ground_1/1 -> HvacTReStatFildForRowSecAtVentSideTopLe_HvacTReStatFildForRowSecAtVentSideTopLe_write/1
  Ground_2/1 -> HvacTReStatFildForRowSecAtVentSideTopRi_HvacTReStatFildForRowSecAtVentSideTopRi_write/1
  Ground_3/1 -> HvacTReStatFildForRowThrdAtFlrLe_HvacTReStatFildForRowThrdAtFlrLe_write/1
  Ground_4/1 -> HvacTReStatFildForRowThrdAtFlrRi_HvacTReStatFildForRowThrdAtFlrRi_write/1
  Ground_5/1 -> HvacTReStatFildForRowThrdAtVentSideTopLe_HvacTReStatFildForRowThrdAtVentSideTopLe_write/1
  Ground_6/1 -> HvacTReStatFildForRowThrdAtVentSideTopRi_HvacTReStatFildForRowThrdAtVentSideTopRi_write/1
  Ground_7/1 -> HvacTFrntStatFildForRowFirstAtDefrstLe_HvacTFrntStatFildForRowFirstAtDefrstLe_write/1
  Ground_8/1 -> HvacTFrntStatFildForRowFirstAtDefrstRi_HvacTFrntStatFildForRowFirstAtDefrstRi_write/1
  Ground_9/1 -> HvacTFrntStatFildForRowFirstAtDefrstSideLe_HvacTFrntStatFildForRowFirstAtDefrstSideLe_write/1
  Ground_10/1 -> HvacTFrntStatFildForRowFirstAtDefrstSideRi_HvacTFrntStatFildForRowFirstAtDefrstSideRi_write/1
  Ground_11/1 -> HvacTFrntStatFildForRowFirstAtFlrLe_HvacTFrntStatFildForRowFirstAtFlrLe_write/1
  Ground_12/1 -> HvacTFrntStatFildForRowFirstAtFlrRi_HvacTFrntStatFildForRowFirstAtFlrRi_write/1
  Ground_13/1 -> HvacTFrntStatFildForRowFirstAtVentLe_HvacTFrntStatFildForRowFirstAtVentLe_write/1
  Ground_14/1 -> HvacTFrntStatFildForRowFirstAtVentRi_HvacTFrntStatFildForRowFirstAtVentRi_write/1
  Ground_15/1 -> HvacTFrntStatFildForRowFirstAtVentSideLe_HvacTFrntStatFildForRowFirstAtVentSideLe_write/1
  Ground_16/1 -> HvacTFrntStatFildForRowFirstAtVentSideRi_HvacTFrntStatFildForRowFirstAtVentSideRi_write/1
  Ground_17/1 -> HvacTFrntStatFildForRowSecAtFlrLe_HvacTFrntStatFildForRowSecAtFlrLe_write/1
  Ground_18/1 -> HvacTFrntStatFildForRowSecAtFlrRi_HvacTFrntStatFildForRowSecAtFlrRi_write/1
  Ground_19/1 -> HvacTFrntStatFildForRowSecAtVentLe_HvacTFrntStatFildForRowSecAtVentLe_write/1
  Ground_20/1 -> HvacTFrntStatFildForRowSecAtVentRi_HvacTFrntStatFildForRowSecAtVentRi_write/1
  Ground_21/1 -> HvacTFrntStatFildForRowSecAtVentSideLe_HvacTFrntStatFildForRowSecAtVentSideLe_write/1
  Ground_22/1 -> HvacTFrntStatFildForRowSecAtVentSideRi_HvacTFrntStatFildForRowSecAtVentSideRi_write/1
